simulink model "Good_creator"  (R2022a)
settings: Solver=FixedStepAuto  StartTime=0.0  StopTime=8  FixedStep=1e-3  RelTol=1e-3
values from neighbouring files (symbols this model uses):
  base = Mb*9.8/4+mw*9.8+0.5*9.8*Mv    (= 46305)    [parameterSetting.m]
  d = 2.6    [parameterSetting.m]
  I = 3055e-8    (= 3.055e-05)    [parameterSetting.m]
  Jv = 3044    [parameterSetting.m]
  kf = 6.67e7    (= 66700000)    [parameterSetting.m]
  Kv = 1.5e6    (= 1500000)    [parameterSetting.m]
  L = beamLength(k)*210    [BuildDataSample.m]    (also in parameterSetting.m = 210)
  m = 7800*(7684e-6)    (= 59.9352)    [parameterSetting.m]
  Mv = 4060/2    (= 2030)    [parameterSetting.m]
  mw = 1670/2    (= 835)    [parameterSetting.m]
  position = 0.55*L    [DatacreatorForChapter4.m]    (also in Figure4_9.m = 94.5; chapter4_3.m = 105; parameterSetting.m = 105)
  v = vkmh/3.6    [BuildDataSample.m]    (also in DatacreatorForChapter4.m = vkmh_set(j)/3.6; Figure4_9.m = vkmh_set(i); chapter4_1.m = vkmh_set(i)/3.6; chapter4_3.m = 13.8888888889)
(part 9 of 78)

== system Good_creator/Subsystem3/Subsystem1/Subsystem2/Subsystem5 ==
  index : Inport
  F : Inport  Port=2
  Add : Sum
  Add1 : Sum
  Constant2 : Constant  Value=2
  Constant6 : Constant
  Constant7 : Constant  Value=2
  Constant8 : Constant  Value=2
  Demux : Demux  Outputs=2
  Mux : Mux  Inputs=2
  Subsystem : Subsystem
  Subsystem1 : Subsystem
  Subsystem2 : Subsystem
  Subsystem4 : Subsystem
  Out1 : Outport
  wires:
  index/1 -> Subsystem/1(imod), Subsystem1/1(imod)
  Mux/1 -> Out1/1
  Constant2/1 -> Subsystem/2(r)
  Constant6/1 -> Subsystem/3(k)
  Constant8/1 -> Subsystem1/3(k)
  Constant7/1 -> Subsystem1/2(r)
  F/1 -> Demux/1
  Demux/1 -> Subsystem/4(F)
  Demux/2 -> Subsystem1/4(F)
  Subsystem/2(theta_n1) -> Subsystem4/2(theta_n1)
  Subsystem/3(theta_n2) -> Subsystem4/3(theta_n2)
  Subsystem/4(theta_n3) -> Subsystem4/4(theta_n3)
  Subsystem/1(An1) -> Subsystem4/1(An1)
  Subsystem/5(Sn) -> Subsystem4/5(Sn)
  Subsystem/6(static_n) -> Subsystem4/6(static_n)
  Subsystem/7(Omiga_bn) -> Subsystem4/7(Omiga_bn)
  Subsystem/8(Omiga_n) -> Subsystem4/8(Omiga_n)
  Subsystem1/1(An1) -> Subsystem2/1(An1)
  Subsystem1/2(theta_n1) -> Subsystem2/2(theta_n1)
  Subsystem1/3(theta_n2) -> Subsystem2/3(theta_n2)
  Subsystem1/4(theta_n3) -> Subsystem2/4(theta_n3)
  Subsystem1/5(Sn) -> Subsystem2/5(Sn)
  Subsystem1/6(static_n) -> Subsystem2/6(static_n)
  Subsystem1/7(Omiga_bn) -> Subsystem2/7(Omiga_bn)
  Subsystem1/8(Omiga_n) -> Subsystem2/8(Omiga_n)
  Subsystem4/1(Out1) -> Add/1
  Subsystem2/1(Out1) -> Add/2
  Subsystem2/2(Out2) -> Add1/2
  Subsystem4/2(Out2) -> Add1/1
  Add/1 -> Mux/1
  Add1/1 -> Mux/2

== system Good_creator/Subsystem3/Subsystem1/Subsystem2/Subsystem6 ==
  index : Inport
  F : Inport  Port=2
  Add : Sum
  Add1 : Sum
  Constant2 : Constant  Value=2
  Constant6 : Constant
  Constant7 : Constant  Value=2
  Constant8 : Constant  Value=2
  Demux : Demux  Outputs=2
  Mux : Mux  Inputs=2
  Subsystem : Subsystem
  Subsystem1 : Subsystem
  Subsystem2 : Subsystem
  Subsystem4 : Subsystem
  Out1 : Outport
  wires:
  Add1/1 -> Mux/2
  Add/1 -> Mux/1
  Subsystem4/2(Out2) -> Add1/1
  Subsystem2/2(Out2) -> Add1/2
  Subsystem2/1(Out1) -> Add/2
  Subsystem4/1(Out1) -> Add/1
  Subsystem1/8(Omiga_n) -> Subsystem2/8(Omiga_n)
  Subsystem1/7(Omiga_bn) -> Subsystem2/7(Omiga_bn)
  Subsystem1/6(static_n) -> Subsystem2/6(static_n)
  Subsystem1/5(Sn) -> Subsystem2/5(Sn)
  Subsystem1/4(theta_n3) -> Subsystem2/4(theta_n3)
  Subsystem1/3(theta_n2) -> Subsystem2/3(theta_n2)
  Subsystem1/2(theta_n1) -> Subsystem2/2(theta_n1)
  Subsystem1/1(An1) -> Subsystem2/1(An1)
  Subsystem/8(Omiga_n) -> Subsystem4/8(Omiga_n)
  Subsystem/7(Omiga_bn) -> Subsystem4/7(Omiga_bn)
  Subsystem/6(static_n) -> Subsystem4/6(static_n)
  Subsystem/5(Sn) -> Subsystem4/5(Sn)
  Subsystem/1(An1) -> Subsystem4/1(An1)
  Subsystem/4(theta_n3) -> Subsystem4/4(theta_n3)
  Subsystem/3(theta_n2) -> Subsystem4/3(theta_n2)
  Subsystem/2(theta_n1) -> Subsystem4/2(theta_n1)
  Demux/2 -> Subsystem1/4(F)
  Demux/1 -> Subsystem/4(F)
  F/1 -> Demux/1
  Constant7/1 -> Subsystem1/2(r)
  Constant8/1 -> Subsystem1/3(k)
  Constant6/1 -> Subsystem/3(k)
  Constant2/1 -> Subsystem/2(r)
  Mux/1 -> Out1/1
  index/1 -> Subsystem1/1(imod), Subsystem/1(imod)

== system Good_creator/Subsystem3/Subsystem1/Subsystem2/Subsystem7 ==
  index : Inport
  F : Inport  Port=2
  Add : Sum
  Add1 : Sum
  Constant2 : Constant  Value=2
  Constant6 : Constant
  Constant7 : Constant  Value=2
  Constant8 : Constant  Value=2
  Demux : Demux  Outputs=2
  Mux : Mux  Inputs=2
  Subsystem : Subsystem
  Subsystem1 : Subsystem
  Subsystem2 : Subsystem
  Subsystem4 : Subsystem
  Out1 : Outport
  wires:
  index/1 -> Subsystem/1(imod), Subsystem1/1(imod)
  Mux/1 -> Out1/1
  Constant2/1 -> Subsystem/2(r)
  Constant6/1 -> Subsystem/3(k)
  Constant8/1 -> Subsystem1/3(k)
  Constant7/1 -> Subsystem1/2(r)
  F/1 -> Demux/1
  Demux/1 -> Subsystem/4(F)
  Demux/2 -> Subsystem1/4(F)
  Subsystem/2(theta_n1) -> Subsystem4/2(theta_n1)
  Subsystem/3(theta_n2) -> Subsystem4/3(theta_n2)
  Subsystem/4(theta_n3) -> Subsystem4/4(theta_n3)
  Subsystem/1(An1) -> Subsystem4/1(An1)
  Subsystem/5(Sn) -> Subsystem4/5(Sn)
  Subsystem/6(static_n) -> Subsystem4/6(static_n)
  Subsystem/7(Omiga_bn) -> Subsystem4/7(Omiga_bn)
  Subsystem/8(Omiga_n) -> Subsystem4/8(Omiga_n)
  Subsystem1/1(An1) -> Subsystem2/1(An1)
  Subsystem1/2(theta_n1) -> Subsystem2/2(theta_n1)
  Subsystem1/3(theta_n2) -> Subsystem2/3(theta_n2)
  Subsystem1/4(theta_n3) -> Subsystem2/4(theta_n3)
  Subsystem1/5(Sn) -> Subsystem2/5(Sn)
  Subsystem1/6(static_n) -> Subsystem2/6(static_n)
  Subsystem1/7(Omiga_bn) -> Subsystem2/7(Omiga_bn)
  Subsystem1/8(Omiga_n) -> Subsystem2/8(Omiga_n)
  Subsystem4/1(Out1) -> Add/1
  Subsystem2/1(Out1) -> Add/2
  Subsystem2/2(Out2) -> Add1/2
  Subsystem4/2(Out2) -> Add1/1
  Add/1 -> Mux/1
  Add1/1 -> Mux/2

== system Good_creator/Subsystem3/Subsystem1/Subsystem2/Subsystem8 ==
  index : Inport
  F : Inport  Port=2
  Add : Sum
  Add1 : Sum
  Constant2 : Constant  Value=2
  Constant6 : Constant
  Constant7 : Constant  Value=2
  Constant8 : Constant  Value=2
  Demux : Demux  Outputs=2
  Mux : Mux  Inputs=2
  Subsystem : Subsystem
  Subsystem1 : Subsystem
  Subsystem2 : Subsystem
  Subsystem4 : Subsystem
  Out1 : Outport
  wires:
  Add1/1 -> Mux/2
  Add/1 -> Mux/1
  Subsystem4/2(Out2) -> Add1/1
  Subsystem2/2(Out2) -> Add1/2
  Subsystem2/1(Out1) -> Add/2
  Subsystem4/1(Out1) -> Add/1
  Subsystem1/8(Omiga_n) -> Subsystem2/8(Omiga_n)
  Subsystem1/7(Omiga_bn) -> Subsystem2/7(Omiga_bn)
  Subsystem1/6(static_n) -> Subsystem2/6(static_n)
  Subsystem1/5(Sn) -> Subsystem2/5(Sn)
  Subsystem1/4(theta_n3) -> Subsystem2/4(theta_n3)
  Subsystem1/3(theta_n2) -> Subsystem2/3(theta_n2)
  Subsystem1/2(theta_n1) -> Subsystem2/2(theta_n1)
  Subsystem1/1(An1) -> Subsystem2/1(An1)
  Subsystem/8(Omiga_n) -> Subsystem4/8(Omiga_n)
  Subsystem/7(Omiga_bn) -> Subsystem4/7(Omiga_bn)
  Subsystem/6(static_n) -> Subsystem4/6(static_n)
  Subsystem/5(Sn) -> Subsystem4/5(Sn)
  Subsystem/1(An1) -> Subsystem4/1(An1)
  Subsystem/4(theta_n3) -> Subsystem4/4(theta_n3)
  Subsystem/3(theta_n2) -> Subsystem4/3(theta_n2)
  Subsystem/2(theta_n1) -> Subsystem4/2(theta_n1)
  Demux/2 -> Subsystem1/4(F)
  Demux/1 -> Subsystem/4(F)
  F/1 -> Demux/1
  Constant7/1 -> Subsystem1/2(r)
  Constant8/1 -> Subsystem1/3(k)
  Constant6/1 -> Subsystem/3(k)
  Constant2/1 -> Subsystem/2(r)
  Mux/1 -> Out1/1
  index/1 -> Subsystem1/1(imod), Subsystem/1(imod)

== system Good_creator/Subsystem3/Subsystem1/Subsystem2/Subsystem9 ==
  index : Inport
  F : Inport  Port=2
  Add : Sum
  Add1 : Sum
  Constant2 : Constant  Value=2
  Constant6 : Constant
  Constant7 : Constant  Value=2
  Constant8 : Constant  Value=2
  Demux : Demux  Outputs=2
  Mux : Mux  Inputs=2
  Subsystem : Subsystem
  Subsystem1 : Subsystem
  Subsystem2 : Subsystem
  Subsystem4 : Subsystem
  Out1 : Outport
  wires:
  index/1 -> Subsystem/1(imod), Subsystem1/1(imod)
  Mux/1 -> Out1/1
  Constant2/1 -> Subsystem/2(r)
  Constant6/1 -> Subsystem/3(k)
  Constant8/1 -> Subsystem1/3(k)
  Constant7/1 -> Subsystem1/2(r)
  F/1 -> Demux/1
  Demux/1 -> Subsystem/4(F)
  Demux/2 -> Subsystem1/4(F)
  Subsystem/2(theta_n1) -> Subsystem4/2(theta_n1)
  Subsystem/3(theta_n2) -> Subsystem4/3(theta_n2)
  Subsystem/4(theta_n3) -> Subsystem4/4(theta_n3)
  Subsystem/1(An1) -> Subsystem4/1(An1)
  Subsystem/5(Sn) -> Subsystem4/5(Sn)
  Subsystem/6(static_n) -> Subsystem4/6(static_n)
  Subsystem/7(Omiga_bn) -> Subsystem4/7(Omiga_bn)
  Subsystem/8(Omiga_n) -> Subsystem4/8(Omiga_n)
  Subsystem1/1(An1) -> Subsystem2/1(An1)
  Subsystem1/2(theta_n1) -> Subsystem2/2(theta_n1)
  Subsystem1/3(theta_n2) -> Subsystem2/3(theta_n2)
  Subsystem1/4(theta_n3) -> Subsystem2/4(theta_n3)
  Subsystem1/5(Sn) -> Subsystem2/5(Sn)
  Subsystem1/6(static_n) -> Subsystem2/6(static_n)
  Subsystem1/7(Omiga_bn) -> Subsystem2/7(Omiga_bn)
  Subsystem1/8(Omiga_n) -> Subsystem2/8(Omiga_n)
  Subsystem4/1(Out1) -> Add/1
  Subsystem2/1(Out1) -> Add/2
  Subsystem2/2(Out2) -> Add1/2
  Subsystem4/2(Out2) -> Add1/1
  Add/1 -> Mux/1
  Add1/1 -> Mux/2

== system Good_creator/Subsystem3/Subsystem1/Subsystem3/Subsystem1 ==
  index : Inport
  F : Inport  Port=2
  Add : Sum
  Add1 : Sum
  Constant2 : Constant  Value=2
  Constant6 : Constant
  Constant7 : Constant  Value=2
  Constant8 : Constant  Value=2
  Demux : Demux  Outputs=2
  Mux : Mux  Inputs=2
  Subsystem : Subsystem
  Subsystem1 : Subsystem
  Subsystem2 : Subsystem
  Subsystem4 : Subsystem
  Out1 : Outport
  wires:
  index/1 -> Subsystem/1(imod), Subsystem1/1(imod)
  Mux/1 -> Out1/1
  Constant2/1 -> Subsystem/2(r)
  Constant6/1 -> Subsystem/3(k)
  Constant8/1 -> Subsystem1/3(k)
  Constant7/1 -> Subsystem1/2(r)
  F/1 -> Demux/1
  Demux/1 -> Subsystem/4(F)
  Demux/2 -> Subsystem1/4(F)
  Subsystem/2(theta_n1) -> Subsystem4/2(theta_n1)
  Subsystem/3(theta_n2) -> Subsystem4/3(theta_n2)
  Subsystem/4(theta_n3) -> Subsystem4/4(theta_n3)
  Subsystem/1(An1) -> Subsystem4/1(An1)
  Subsystem/5(Sn) -> Subsystem4/5(Sn)
  Subsystem/6(static_n) -> Subsystem4/6(static_n)
  Subsystem/7(Omiga_bn) -> Subsystem4/7(Omiga_bn)
  Subsystem/8(Omiga_n) -> Subsystem4/8(Omiga_n)
  Subsystem1/1(An1) -> Subsystem2/1(An1)
  Subsystem1/2(theta_n1) -> Subsystem2/2(theta_n1)
  Subsystem1/3(theta_n2) -> Subsystem2/3(theta_n2)
  Subsystem1/4(theta_n3) -> Subsystem2/4(theta_n3)
  Subsystem1/5(Sn) -> Subsystem2/5(Sn)
  Subsystem1/6(static_n) -> Subsystem2/6(static_n)
  Subsystem1/7(Omiga_bn) -> Subsystem2/7(Omiga_bn)
  Subsystem1/8(Omiga_n) -> Subsystem2/8(Omiga_n)
  Subsystem4/1(Out1) -> Add/1
  Subsystem2/1(Out1) -> Add/2
  Subsystem2/2(Out2) -> Add1/2
  Subsystem4/2(Out2) -> Add1/1
  Add/1 -> Mux/1
  Add1/1 -> Mux/2

== system Good_creator/Subsystem3/Subsystem1/Subsystem3/Subsystem10 ==
  index : Inport
  F : Inport  Port=2
  Add : Sum
  Add1 : Sum
  Constant2 : Constant  Value=2
  Constant6 : Constant
  Constant7 : Constant  Value=2
  Constant8 : Constant  Value=2
  Demux : Demux  Outputs=2
  Mux : Mux  Inputs=2
  Subsystem : Subsystem
  Subsystem1 : Subsystem
  Subsystem2 : Subsystem
  Subsystem4 : Subsystem
  Out1 : Outport
  wires:
  index/1 -> Subsystem/1(imod), Subsystem1/1(imod)
  Mux/1 -> Out1/1
  Constant2/1 -> Subsystem/2(r)
  Constant6/1 -> Subsystem/3(k)
  Constant8/1 -> Subsystem1/3(k)
  Constant7/1 -> Subsystem1/2(r)
  F/1 -> Demux/1
  Demux/1 -> Subsystem/4(F)
  Demux/2 -> Subsystem1/4(F)
  Subsystem/2(theta_n1) -> Subsystem4/2(theta_n1)
  Subsystem/3(theta_n2) -> Subsystem4/3(theta_n2)
  Subsystem/4(theta_n3) -> Subsystem4/4(theta_n3)
  Subsystem/1(An1) -> Subsystem4/1(An1)
  Subsystem/5(Sn) -> Subsystem4/5(Sn)
  Subsystem/6(static_n) -> Subsystem4/6(static_n)
  Subsystem/7(Omiga_bn) -> Subsystem4/7(Omiga_bn)
  Subsystem/8(Omiga_n) -> Subsystem4/8(Omiga_n)
  Subsystem1/1(An1) -> Subsystem2/1(An1)
  Subsystem1/2(theta_n1) -> Subsystem2/2(theta_n1)
  Subsystem1/3(theta_n2) -> Subsystem2/3(theta_n2)
  Subsystem1/4(theta_n3) -> Subsystem2/4(theta_n3)
  Subsystem1/5(Sn) -> Subsystem2/5(Sn)
  Subsystem1/6(static_n) -> Subsystem2/6(static_n)
  Subsystem1/7(Omiga_bn) -> Subsystem2/7(Omiga_bn)
  Subsystem1/8(Omiga_n) -> Subsystem2/8(Omiga_n)
  Subsystem4/1(Out1) -> Add/1
  Subsystem2/1(Out1) -> Add/2
  Subsystem2/2(Out2) -> Add1/2
  Subsystem4/2(Out2) -> Add1/1
  Add/1 -> Mux/1
  Add1/1 -> Mux/2

== system Good_creator/Subsystem3/Subsystem1/Subsystem3/Subsystem11 ==
  index : Inport
  F : Inport  Port=2
  Add : Sum
  Add1 : Sum
  Constant2 : Constant  Value=2
  Constant6 : Constant
  Constant7 : Constant  Value=2
  Constant8 : Constant  Value=2
  Demux : Demux  Outputs=2
  Mux : Mux  Inputs=2
  Subsystem : Subsystem
  Subsystem1 : Subsystem
  Subsystem2 : Subsystem
  Subsystem4 : Subsystem
  Out1 : Outport
  wires:
  Add1/1 -> Mux/2
  Add/1 -> Mux/1
  Subsystem4/2(Out2) -> Add1/1
  Subsystem2/2(Out2) -> Add1/2
  Subsystem2/1(Out1) -> Add/2
  Subsystem4/1(Out1) -> Add/1
  Subsystem1/8(Omiga_n) -> Subsystem2/8(Omiga_n)
  Subsystem1/7(Omiga_bn) -> Subsystem2/7(Omiga_bn)
  Subsystem1/6(static_n) -> Subsystem2/6(static_n)
  Subsystem1/5(Sn) -> Subsystem2/5(Sn)
  Subsystem1/4(theta_n3) -> Subsystem2/4(theta_n3)
  Subsystem1/3(theta_n2) -> Subsystem2/3(theta_n2)
  Subsystem1/2(theta_n1) -> Subsystem2/2(theta_n1)
  Subsystem1/1(An1) -> Subsystem2/1(An1)
  Subsystem/8(Omiga_n) -> Subsystem4/8(Omiga_n)
  Subsystem/7(Omiga_bn) -> Subsystem4/7(Omiga_bn)
  Subsystem/6(static_n) -> Subsystem4/6(static_n)
  Subsystem/5(Sn) -> Subsystem4/5(Sn)
  Subsystem/1(An1) -> Subsystem4/1(An1)
  Subsystem/4(theta_n3) -> Subsystem4/4(theta_n3)
  Subsystem/3(theta_n2) -> Subsystem4/3(theta_n2)
  Subsystem/2(theta_n1) -> Subsystem4/2(theta_n1)
  Demux/2 -> Subsystem1/4(F)
  Demux/1 -> Subsystem/4(F)
  F/1 -> Demux/1
  Constant7/1 -> Subsystem1/2(r)
  Constant8/1 -> Subsystem1/3(k)
  Constant6/1 -> Subsystem/3(k)
  Constant2/1 -> Subsystem/2(r)
  Mux/1 -> Out1/1
  index/1 -> Subsystem1/1(imod), Subsystem/1(imod)

== system Good_creator/Subsystem3/Subsystem1/Subsystem3/Subsystem12 ==
  index : Inport
  F : Inport  Port=2
  Add : Sum
  Add1 : Sum
  Constant2 : Constant  Value=2
  Constant6 : Constant
  Constant7 : Constant  Value=2
  Constant8 : Constant  Value=2
  Demux : Demux  Outputs=2
  Mux : Mux  Inputs=2
  Subsystem : Subsystem
  Subsystem1 : Subsystem
  Subsystem2 : Subsystem
  Subsystem4 : Subsystem
  Out1 : Outport
  wires:
  index/1 -> Subsystem/1(imod), Subsystem1/1(imod)
  Mux/1 -> Out1/1
  Constant2/1 -> Subsystem/2(r)
  Constant6/1 -> Subsystem/3(k)
  Constant8/1 -> Subsystem1/3(k)
  Constant7/1 -> Subsystem1/2(r)
  F/1 -> Demux/1
  Demux/1 -> Subsystem/4(F)
  Demux/2 -> Subsystem1/4(F)
  Subsystem/2(theta_n1) -> Subsystem4/2(theta_n1)
  Subsystem/3(theta_n2) -> Subsystem4/3(theta_n2)
  Subsystem/4(theta_n3) -> Subsystem4/4(theta_n3)
  Subsystem/1(An1) -> Subsystem4/1(An1)
  Subsystem/5(Sn) -> Subsystem4/5(Sn)
  Subsystem/6(static_n) -> Subsystem4/6(static_n)
  Subsystem/7(Omiga_bn) -> Subsystem4/7(Omiga_bn)
  Subsystem/8(Omiga_n) -> Subsystem4/8(Omiga_n)
  Subsystem1/1(An1) -> Subsystem2/1(An1)
  Subsystem1/2(theta_n1) -> Subsystem2/2(theta_n1)
  Subsystem1/3(theta_n2) -> Subsystem2/3(theta_n2)
  Subsystem1/4(theta_n3) -> Subsystem2/4(theta_n3)
  Subsystem1/5(Sn) -> Subsystem2/5(Sn)
  Subsystem1/6(static_n) -> Subsystem2/6(static_n)
  Subsystem1/7(Omiga_bn) -> Subsystem2/7(Omiga_bn)
  Subsystem1/8(Omiga_n) -> Subsystem2/8(Omiga_n)
  Subsystem4/1(Out1) -> Add/1
  Subsystem2/1(Out1) -> Add/2
  Subsystem2/2(Out2) -> Add1/2
  Subsystem4/2(Out2) -> Add1/1
  Add/1 -> Mux/1
  Add1/1 -> Mux/2

== system Good_creator/Subsystem3/Subsystem1/Subsystem3/Subsystem13 ==
  index : Inport
  F : Inport  Port=2
  Add : Sum
  Add1 : Sum
  Constant2 : Constant  Value=2
  Constant6 : Constant
  Constant7 : Constant  Value=2
  Constant8 : Constant  Value=2
  Demux : Demux  Outputs=2
  Mux : Mux  Inputs=2
  Subsystem : Subsystem
  Subsystem1 : Subsystem
  Subsystem2 : Subsystem
  Subsystem4 : Subsystem
  Out1 : Outport
  wires:
  index/1 -> Subsystem/1(imod), Subsystem1/1(imod)
  Mux/1 -> Out1/1
  Constant2/1 -> Subsystem/2(r)
  Constant6/1 -> Subsystem/3(k)
  Constant8/1 -> Subsystem1/3(k)
  Constant7/1 -> Subsystem1/2(r)
  F/1 -> Demux/1
  Demux/1 -> Subsystem/4(F)
  Demux/2 -> Subsystem1/4(F)
  Subsystem/2(theta_n1) -> Subsystem4/2(theta_n1)
  Subsystem/3(theta_n2) -> Subsystem4/3(theta_n2)
  Subsystem/4(theta_n3) -> Subsystem4/4(theta_n3)
  Subsystem/1(An1) -> Subsystem4/1(An1)
  Subsystem/5(Sn) -> Subsystem4/5(Sn)
  Subsystem/6(static_n) -> Subsystem4/6(static_n)
  Subsystem/7(Omiga_bn) -> Subsystem4/7(Omiga_bn)
  Subsystem/8(Omiga_n) -> Subsystem4/8(Omiga_n)
  Subsystem1/1(An1) -> Subsystem2/1(An1)
  Subsystem1/2(theta_n1) -> Subsystem2/2(theta_n1)
  Subsystem1/3(theta_n2) -> Subsystem2/3(theta_n2)
  Subsystem1/4(theta_n3) -> Subsystem2/4(theta_n3)
  Subsystem1/5(Sn) -> Subsystem2/5(Sn)
  Subsystem1/6(static_n) -> Subsystem2/6(static_n)
  Subsystem1/7(Omiga_bn) -> Subsystem2/7(Omiga_bn)
  Subsystem1/8(Omiga_n) -> Subsystem2/8(Omiga_n)
  Subsystem4/1(Out1) -> Add/1
  Subsystem2/1(Out1) -> Add/2
  Subsystem2/2(Out2) -> Add1/2
  Subsystem4/2(Out2) -> Add1/1
  Add/1 -> Mux/1
  Add1/1 -> Mux/2

== system Good_creator/Subsystem3/Subsystem1/Subsystem3/Subsystem14 ==
  index : Inport
  F : Inport  Port=2
  Add : Sum
  Add1 : Sum
  Constant2 : Constant  Value=2
  Constant6 : Constant
  Constant7 : Constant  Value=2
  Constant8 : Constant  Value=2
  Demux : Demux  Outputs=2
  Mux : Mux  Inputs=2
  Subsystem : Subsystem
  Subsystem1 : Subsystem
  Subsystem2 : Subsystem
  Subsystem4 : Subsystem
  Out1 : Outport
  wires:
  Add1/1 -> Mux/2
  Add/1 -> Mux/1
  Subsystem4/2(Out2) -> Add1/1
  Subsystem2/2(Out2) -> Add1/2
  Subsystem2/1(Out1) -> Add/2
  Subsystem4/1(Out1) -> Add/1
  Subsystem1/8(Omiga_n) -> Subsystem2/8(Omiga_n)
  Subsystem1/7(Omiga_bn) -> Subsystem2/7(Omiga_bn)
  Subsystem1/6(static_n) -> Subsystem2/6(static_n)
  Subsystem1/5(Sn) -> Subsystem2/5(Sn)
  Subsystem1/4(theta_n3) -> Subsystem2/4(theta_n3)
  Subsystem1/3(theta_n2) -> Subsystem2/3(theta_n2)
  Subsystem1/2(theta_n1) -> Subsystem2/2(theta_n1)
  Subsystem1/1(An1) -> Subsystem2/1(An1)
  Subsystem/8(Omiga_n) -> Subsystem4/8(Omiga_n)
  Subsystem/7(Omiga_bn) -> Subsystem4/7(Omiga_bn)
  Subsystem/6(static_n) -> Subsystem4/6(static_n)
  Subsystem/5(Sn) -> Subsystem4/5(Sn)
  Subsystem/1(An1) -> Subsystem4/1(An1)
  Subsystem/4(theta_n3) -> Subsystem4/4(theta_n3)
  Subsystem/3(theta_n2) -> Subsystem4/3(theta_n2)
  Subsystem/2(theta_n1) -> Subsystem4/2(theta_n1)
  Demux/2 -> Subsystem1/4(F)
  Demux/1 -> Subsystem/4(F)
  F/1 -> Demux/1
  Constant7/1 -> Subsystem1/2(r)
  Constant8/1 -> Subsystem1/3(k)
  Constant6/1 -> Subsystem/3(k)
  Constant2/1 -> Subsystem/2(r)
  Mux/1 -> Out1/1
  index/1 -> Subsystem1/1(imod), Subsystem/1(imod)

== system Good_creator/Subsystem3/Subsystem1/Subsystem3/Subsystem15 ==
  index : Inport
  F : Inport  Port=2
  Add : Sum
  Add1 : Sum
  Constant2 : Constant  Value=2
  Constant6 : Constant
  Constant7 : Constant  Value=2
  Constant8 : Constant  Value=2
  Demux : Demux  Outputs=2
  Mux : Mux  Inputs=2
  Subsystem : Subsystem
  Subsystem1 : Subsystem
  Subsystem2 : Subsystem
  Subsystem4 : Subsystem
  Out1 : Outport
  wires:
  index/1 -> Subsystem/1(imod), Subsystem1/1(imod)
  Mux/1 -> Out1/1
  Constant2/1 -> Subsystem/2(r)
  Constant6/1 -> Subsystem/3(k)
  Constant8/1 -> Subsystem1/3(k)
  Constant7/1 -> Subsystem1/2(r)
  F/1 -> Demux/1
  Demux/1 -> Subsystem/4(F)
  Demux/2 -> Subsystem1/4(F)
  Subsystem/2(theta_n1) -> Subsystem4/2(theta_n1)
  Subsystem/3(theta_n2) -> Subsystem4/3(theta_n2)
  Subsystem/4(theta_n3) -> Subsystem4/4(theta_n3)
  Subsystem/1(An1) -> Subsystem4/1(An1)
  Subsystem/5(Sn) -> Subsystem4/5(Sn)
  Subsystem/6(static_n) -> Subsystem4/6(static_n)
  Subsystem/7(Omiga_bn) -> Subsystem4/7(Omiga_bn)
  Subsystem/8(Omiga_n) -> Subsystem4/8(Omiga_n)
  Subsystem1/1(An1) -> Subsystem2/1(An1)
  Subsystem1/2(theta_n1) -> Subsystem2/2(theta_n1)
  Subsystem1/3(theta_n2) -> Subsystem2/3(theta_n2)
  Subsystem1/4(theta_n3) -> Subsystem2/4(theta_n3)
  Subsystem1/5(Sn) -> Subsystem2/5(Sn)
  Subsystem1/6(static_n) -> Subsystem2/6(static_n)
  Subsystem1/7(Omiga_bn) -> Subsystem2/7(Omiga_bn)
  Subsystem1/8(Omiga_n) -> Subsystem2/8(Omiga_n)
  Subsystem4/1(Out1) -> Add/1
  Subsystem2/1(Out1) -> Add/2
  Subsystem2/2(Out2) -> Add1/2
  Subsystem4/2(Out2) -> Add1/1
  Add/1 -> Mux/1
  Add1/1 -> Mux/2

== system Good_creator/Subsystem3/Subsystem1/Subsystem3/Subsystem16 ==
  index : Inport
  F : Inport  Port=2
  Add : Sum
  Add1 : Sum
  Constant2 : Constant  Value=2
  Constant6 : Constant
  Constant7 : Constant  Value=2
  Constant8 : Constant  Value=2
  Demux : Demux  Outputs=2
  Mux : Mux  Inputs=2
  Subsystem : Subsystem
  Subsystem1 : Subsystem
  Subsystem2 : Subsystem
  Subsystem4 : Subsystem
  Out1 : Outport
  wires:
  Add1/1 -> Mux/2
  Add/1 -> Mux/1
  Subsystem4/2(Out2) -> Add1/1
  Subsystem2/2(Out2) -> Add1/2
  Subsystem2/1(Out1) -> Add/2
  Subsystem4/1(Out1) -> Add/1
  Subsystem1/8(Omiga_n) -> Subsystem2/8(Omiga_n)
  Subsystem1/7(Omiga_bn) -> Subsystem2/7(Omiga_bn)
  Subsystem1/6(static_n) -> Subsystem2/6(static_n)
  Subsystem1/5(Sn) -> Subsystem2/5(Sn)
  Subsystem1/4(theta_n3) -> Subsystem2/4(theta_n3)
  Subsystem1/3(theta_n2) -> Subsystem2/3(theta_n2)
  Subsystem1/2(theta_n1) -> Subsystem2/2(theta_n1)
  Subsystem1/1(An1) -> Subsystem2/1(An1)
  Subsystem/8(Omiga_n) -> Subsystem4/8(Omiga_n)
  Subsystem/7(Omiga_bn) -> Subsystem4/7(Omiga_bn)
  Subsystem/6(static_n) -> Subsystem4/6(static_n)
  Subsystem/5(Sn) -> Subsystem4/5(Sn)
  Subsystem/1(An1) -> Subsystem4/1(An1)
  Subsystem/4(theta_n3) -> Subsystem4/4(theta_n3)
  Subsystem/3(theta_n2) -> Subsystem4/3(theta_n2)
  Subsystem/2(theta_n1) -> Subsystem4/2(theta_n1)
  Demux/2 -> Subsystem1/4(F)
  Demux/1 -> Subsystem/4(F)
  F/1 -> Demux/1
  Constant7/1 -> Subsystem1/2(r)
  Constant8/1 -> Subsystem1/3(k)
  Constant6/1 -> Subsystem/3(k)
  Constant2/1 -> Subsystem/2(r)
  Mux/1 -> Out1/1
  index/1 -> Subsystem1/1(imod), Subsystem/1(imod)

== system Good_creator/Subsystem3/Subsystem1/Subsystem3/Subsystem17 ==
  index : Inport
  F : Inport  Port=2
  Add : Sum
  Add1 : Sum
  Constant2 : Constant  Value=2
  Constant6 : Constant
  Constant7 : Constant  Value=2
  Constant8 : Constant  Value=2
  Demux : Demux  Outputs=2
  Mux : Mux  Inputs=2
  Subsystem : Subsystem
  Subsystem1 : Subsystem
  Subsystem2 : Subsystem
  Subsystem4 : Subsystem
  Out1 : Outport
  wires:
  Add1/1 -> Mux/2
  Add/1 -> Mux/1
  Subsystem4/2(Out2) -> Add1/1
  Subsystem2/2(Out2) -> Add1/2
  Subsystem2/1(Out1) -> Add/2
  Subsystem4/1(Out1) -> Add/1
  Subsystem1/8(Omiga_n) -> Subsystem2/8(Omiga_n)
  Subsystem1/7(Omiga_bn) -> Subsystem2/7(Omiga_bn)
  Subsystem1/6(static_n) -> Subsystem2/6(static_n)
  Subsystem1/5(Sn) -> Subsystem2/5(Sn)
  Subsystem1/4(theta_n3) -> Subsystem2/4(theta_n3)
  Subsystem1/3(theta_n2) -> Subsystem2/3(theta_n2)
  Subsystem1/2(theta_n1) -> Subsystem2/2(theta_n1)
  Subsystem1/1(An1) -> Subsystem2/1(An1)
  Subsystem/8(Omiga_n) -> Subsystem4/8(Omiga_n)
  Subsystem/7(Omiga_bn) -> Subsystem4/7(Omiga_bn)
  Subsystem/6(static_n) -> Subsystem4/6(static_n)
  Subsystem/5(Sn) -> Subsystem4/5(Sn)
  Subsystem/1(An1) -> Subsystem4/1(An1)
  Subsystem/4(theta_n3) -> Subsystem4/4(theta_n3)
  Subsystem/3(theta_n2) -> Subsystem4/3(theta_n2)
  Subsystem/2(theta_n1) -> Subsystem4/2(theta_n1)
  Demux/2 -> Subsystem1/4(F)
  Demux/1 -> Subsystem/4(F)
  F/1 -> Demux/1
  Constant7/1 -> Subsystem1/2(r)
  Constant8/1 -> Subsystem1/3(k)
  Constant6/1 -> Subsystem/3(k)
  Constant2/1 -> Subsystem/2(r)
  Mux/1 -> Out1/1
  index/1 -> Subsystem1/1(imod), Subsystem/1(imod)
